simulink model "MCVT_Integration"  (R2020a)
settings: Solver=ode8  StartTime=0.0  StopTime=T_end  FixedStep=dt  RelTol=1e-3  EnableMultiTasking=on
values from neighbouring files (symbols this model uses):
  assumed_power_voltage = 1.25e2    (= 125)    [MCVT_Input_File.m]    (also in MCVT_Run_QuickCheck.m)
  battery_power_toggle = 1    [MCVT_Input_File.m]    (also in MCVT_Run_QuickCheck.m)
  battery_trickle_charge_param = 0.1    [MCVT_Input_File.m]    (also in MCVT_Run_QuickCheck.m)
  battery_trickle_leakage_param = 0.01    [MCVT_Input_File.m]    (also in MCVT_Run_QuickCheck.m)
  damage_case_id = 3    [MCVT_Input_File.m]    (also in MCVT_Run_QuickCheck.m = 1)
  dt = 1/fs    (= 0.001)    [MCVT_Run_QuickCheck.m]    (also in MCVT_Sim_Set.m)
  dt_2 = 1/fs_2    (= 0.001)    [MCVT_Run_QuickCheck.m]    (also in MCVT_Sim_Set.m)
  dt_3 = 1/fs_3    (= 0.001)    [MCVT_Run_QuickCheck.m]    (also in MCVT_Sim_Set.m)
  dt_5 = 1/fs_5    (= 0.005)    [MCVT_Run_QuickCheck.m]    (also in MCVT_Sim_Set.m)
  dt_8 = 1/fs_8    (= 0.05)    [MCVT_Run_QuickCheck.m]    (also in MCVT_Sim_Set.m)
  dt_9 = 1/fs_9    (= 0.001)    [MCVT_Run_QuickCheck.m]    (also in MCVT_Sim_Set.m)
  dust_met_duration = 3    [MCVT_Input_File.m]    (also in MCVT_Run_QuickCheck.m = 2)
  ECLSS_indoor_set_temperature = 298.15    [MCVT_Input_File.m]    (also in MCVT_Run_QuickCheck.m)
  energy_store_max_o = 150    [MCVT_Input_File.m]    (also in MCVT_Run_QuickCheck.m)
  energy_store_o = 120    [MCVT_Input_File.m]    (also in MCVT_Run_QuickCheck.m)
  fs = 1000    [MCVT_Run_QuickCheck.m]    (also in MCVT_Sim_Set.m)
  gain_charge_param = 1    [MCVT_Input_File.m]    (also in MCVT_Run_QuickCheck.m)
  ground_temperature = 373    [MCVT_Habitat_Design_Input_File.m]    (also in MCVT_Run_QuickCheck.m)
  I_max_charge = 800    [MCVT_Input_File.m]    (also in MCVT_Run_QuickCheck.m)
  I_max_discharge = 800    [MCVT_Input_File.m]    (also in MCVT_Run_QuickCheck.m)
  initial_pressure = 1e5    (= 100000)    [MCVT_Input_File.m]    (also in MCVT_Run_QuickCheck.m)
  initial_temperature = 295    [MCVT_Input_File.m]    (also in MCVT_Run_QuickCheck.m)
  interior_leak_rate = 0.00415    [MCVT_Input_File.m]    (also in MCVT_Run_QuickCheck.m)
  launch_dust_accum_start_time = 10    [MCVT_Input_File.m]    (also in MCVT_Run_QuickCheck.m = 2)
  launch_dust_accum_stop_time = 12    [MCVT_Input_File.m]    (also in MCVT_Run_QuickCheck.m = 3)
  meteorite_impact_time = 2    [MCVT_Input_File.m]    (also in MCVT_Run_QuickCheck.m = 1)
  nuclear_power_toggle = 1    [MCVT_Input_File.m]    (also in MCVT_Run_QuickCheck.m)
  print_interval = 30    [MCVT_Input_File.m]
  SOC_lower_thres = 0.5    [MCVT_Input_File.m]    (also in MCVT_Run_QuickCheck.m)
  SOC_upper_thres = 0.9    [MCVT_Input_File.m]    (also in MCVT_Run_QuickCheck.m)
  solar_power_toggle = 1    [MCVT_Input_File.m]    (also in MCVT_Run_QuickCheck.m)
  space_temperature = 4    [MCVT_Habitat_Design_Input_File.m]    (also in MCVT_Run_QuickCheck.m)
  Start_angle = 90    [MCVT_Input_File.m]    (also in MCVT_Run_QuickCheck.m)
undefined symbols (not defined in the file or in its folder): A_flow_restrictor, ACTIVITY_LOOKUP_TABLE, air, Aref, Bmap, Bmap2, Bmap3, comms, DiscIntNeverNeededParam, DmgSPL, dust_parameters, ECLSS_dust_damage_repair_time, ECLSS_heater_capacity, ECLSS_IPS_restrictor, ECLSS_IPS_setpointPressure, ECLSS_paint_damage_repair_time, ECLSS_radiatorLoop_discreteNumber, ECLSS_Vst, emptyActivity, expected_heat_add, FI, habitat, HIEM2SMM, HIEM2STM, HIEMDamageElements, impact_numbering, initial_SPLT_damage_index, input_air, input_Cond, input_CP, input_discretization, input_EV, input_Evap, input_radiatorLoop, K_ECLSS_dust_single, K_ECLSS_paint_single, K_Structure_damage_single, Kref, kW_to_W, meteoroidDustDep, mFRF, ndelays, nominalDustDep, noverlap, nuclear_toggle_gain, output_limits, panel_paint0, Ps0, reference_temperature, RepairTime_ECLSS_factor, Sensor_Location_SMM, SMM_T0, SMM_T1, SMM_T2, SMM_T3, SMM_T4, SMM_T4_0, SMM_T4_1, SMM_T4_2, solar_FDD_K ... (+73)
libraries: neural x350, simulink x39, xpcutilitieslib x7, xpcrtudplib x7, dspstat3 x1
(part 6 of 43)

== system MCVT_Integration/Sys2 - Structural System/Sys2_Structural_System/Structural Thermal Model 2.2/STM Temperature Sensors/ Resistance Temperature Detector2 ==
  Physical Interdependency - IN : Inport
  Switch Control : Inport  Port=2
  Power_Supply (kW) : Inport  Port=3
  Power Consumption : MATLAB Function  TreatAsAtomicUnit=on
  R = U/I : Gain  Gain=1/0.001
  R vs T : MATLAB Function  TreatAsAtomicUnit=on
  RTD with Self-heat Error : Subsystem
  RTD without Self-heat Error : Subsystem
  Self-heat Calculation : Subsystem
  Switch ON/OFF Control Block : Subsystem
  T vs R : MATLAB Function  TreatAsAtomicUnit=on
  Physical Interdependency - OUT : Outport  VectorParamsAs1DForOutWhenUnconnected=off
  Cyber Interdependency - OUT : Outport  Port=2  VectorParamsAs1DForOutWhenUnconnected=off
  Power_Usage (kW) : Outport  Port=3
  wires:
  T vs R/1(R) -> RTD without Self-heat Error/1(Resistance Change without Error), Self-heat Calculation/2(Resistance Change without Error)
  R vs T/1(T) -> Cyber Interdependency - OUT/1
  RTD with Self-heat Error/1(Voltage Output with Error) -> R = U/I/1
  Self-heat Calculation/1(Resistance Change with Error) -> RTD with Self-heat Error/1(Resistance Change with Error)
  R = U/I/1 -> R vs T/1(R)
  RTD without Self-heat Error/1(Current in Circuit) -> Self-heat Calculation/3(Current in Circuit)
  Switch Control/1 -> Switch ON/OFF Control Block/1(In1)
  Physical Interdependency - IN/1 -> Physical Interdependency - OUT/1, Switch ON/OFF Control Block/2(In2)
  Power Consumption/1(kWUsage3) -> Power_Usage (kW)/1
  Switch ON/OFF Control Block/1(Out1) -> Power Consumption/2(Switch), T vs R/1(T), Self-heat Calculation/1(Real Temperature)
  Power_Supply (kW)/1 -> Power Consumption/1(Supply)

== system MCVT_Integration/Sys3 - Power System/Power Generation Subsystem1/Nuclear Power Generation/SP-100 Power Generation/	Power Generation ==
  Disturbance : Inport
  Sub3_In_Dust_Accumulation (mg/cm^2) : Inport  Port=2
  Interpolation : Subsystem
  Nominal Power : Subsystem
  Power Generation : Subsystem
  Sensor Models : Subsystem
  Cyber Output SP100 : Outport
  Physical Output SP100 : Outport  Port=2
  Heat Rejection Fraction of Nominal : Outport  Port=3
  Measured Temp Values Nominal (K) : Outport  Port=4
  Power nominal (kW) : Outport  Port=5
  wires:
  [cyb_output1] -> Cyber Output SP100/1
  Disturbance/1 -> [disturbance]
  Power Generation/1(Physical Output) -> [phys_out]
  [phys_out1] -> Physical Output SP100/1
  Sub3_In_Dust_Accumulation (mg/cm^2)/1 -> [dust_accum4]
  [phys_input] -> Sensor Models/1(Physical Input)
  [tc1_interp] -> Sensor Models/3(tc1)
  [power_interp] -> Sensor Models/2(power (kW))
  [temp_flux] -> Interpolation/2(temp flux)
  [HRF_nom] -> Heat Rejection Fraction of Nominal/1
  [flow] -> Nominal Power/3(flow)
  [meas_nom] -> Measured Temp Values Nominal (K)/1
  [nom_vals] -> Nominal Power/1(nominal values)
  [power_nom1] -> Power nominal (kW)/1
  [disturbance] -> Power Generation/1(Disturbance Input)
  [dust_accum4] -> Power Generation/2(Sub3_In_Dust_Accumulation (mg/cm^2))
  [phys_out] -> Interpolation/1(Physical Output)
  Power Generation/2(temp flux) -> [temp_flux]
  [temp_flux] -> Nominal Power/2(temp flux)
  Power Generation/3(Heat Rejection Fraction of Nominal) -> [HRF_nom]
  Power Generation/4(flow) -> [flow]
  Power Generation/5(nominal values) -> [nom_vals]
  Nominal Power/1(Measured Temp Values Nominal (K)) -> [meas_nom]
  Nominal Power/2(power nominal (kW)) -> [power_nom1]
  Sensor Models/1(Cyber Output) -> [cyb_output1]
  Sensor Models/2(Physical Output SP100) -> [phys_out1]
  Interpolation/1(Physical Input) -> [phys_input]
  Interpolation/2(Tc1_interp (K)) -> [tc1_interp]
  Interpolation/3(Power_interp (kW)) -> [power_interp]

== system MCVT_Integration/Sys3 - Power System/Power Generation Subsystem1/Nuclear Power Generation/SP-100 Power Generation/FDD Iterative Online Residual Analysis1 ==
  Physical Output SP100 : Inport
  power nominal (kW) : Inport  Port=2
  Sub3_In_Intervention : Inport  Port=3
  " Digital Twin of SP100" : Subsystem
  Cyber Output : Outport
  wires:
  " Digital Twin of SP100"/1(Cyber Output FDD) -> Cyber Output/1
  power nominal (kW)/1 -> " Digital Twin of SP100"/2(power nominal (kW))
  Physical Output SP100/1 -> " Digital Twin of SP100"/1(Physical Output SP100)
  Sub3_In_Intervention/1 -> " Digital Twin of SP100"/3(Sub3_In_Intervention)

== system MCVT_Integration/Sys3 - Power System/Power Generation Subsystem1/Power Distribution and Storage System/Subsystem2/Dummy Electrical Power Consuming Equipment3 ==
  XX_In_Power_Available : Inport
  MATLAB Function : MATLAB Function  TreatAsAtomicUnit=on
  Power Draw Desired : Memory  InitialCondition=50
  p_3_desired : Outport
  wires:
  Power Draw Desired/1 -> p_3_desired/1
  MATLAB Function/1(p_desired) -> Power Draw Desired/1
  XX_In_Power_Available/1 -> MATLAB Function/1(p_avail)

== system MCVT_Integration/Sys8 - Interior Environment/Habitat Interior Environment Model/Temperature Sensors/ Resistance Temperature Detector/RTD with Self-heat Error ==
  Resistance Change with Error : Inport
  " Current in Circuit" : Constant  Value=0.001
  MATLAB Function : MATLAB Function  TreatAsAtomicUnit=on
  Voltage Output with Error : Outport  VectorParamsAs1DForOutWhenUnconnected=off
  wires:
  Resistance Change with Error/1 -> MATLAB Function/2(R)
  MATLAB Function/1(U) -> Voltage Output with Error/1
  " Current in Circuit"/1 -> MATLAB Function/1(I)

== system MCVT_Integration/Sys8 - Interior Environment/Habitat Interior Environment Model/Temperature Sensors/ Resistance Temperature Detector/RTD without Self-heat Error ==
  Resistance Change without Error : Inport
  " Current in Circuit" : Constant  Value=0.001
  MATLAB Function : MATLAB Function  TreatAsAtomicUnit=on
  Current in Circuit : Outport  VectorParamsAs1DForOutWhenUnconnected=off
  Voltage Output without Error : Outport  Port=2  VectorParamsAs1DForOutWhenUnconnected=off
  wires:
  Resistance Change without Error/1 -> MATLAB Function/2(R)
  MATLAB Function/2(U) -> Voltage Output without Error/1
  MATLAB Function/1(I) -> Current in Circuit/1
  " Current in Circuit"/1 -> MATLAB Function/1("I ")

== system MCVT_Integration/Sys8 - Interior Environment/Habitat Interior Environment Model/Temperature Sensors/ Resistance Temperature Detector/Self-heat Calculation ==
  note: Power = I 2 *R
  Real Temperature : Inport
  Resistance Change without Error : Inport  Port=2
  Current in Circuit : Inport  Port=3
  "  T vs R " : MATLAB Function  TreatAsAtomicUnit=on
  Add1 : Sum
  Divide1 : Product  Inputs=*/
  Gain : Gain  Gain=1000
  Product : Product
  Self Heating Constant (mW/℃) : Constant  Value=50
  Square : Math  Operator=square  SignedPower=on  SaturateOnIntegerOverflow=on
  Resistance Change with Error : Outport  VectorParamsAs1DForOutWhenUnconnected=off
  Self-heat Error : Outport  Port=2  VectorParamsAs1DForOutWhenUnconnected=off
  wires:
  Self Heating Constant (mW/℃)/1 -> Divide1/2
  Product/1 -> Divide1/1
  Add1/1 -> "  T vs R "/1(T)
  Divide1/1 -> Self-heat Error/1, Add1/2
  Resistance Change without Error/1 -> Product/1
  "  T vs R "/1(R) -> Resistance Change with Error/1
  Real Temperature/1 -> Add1/1
  Gain/1 -> Square/1
  Square/1 -> Product/2
  Current in Circuit/1 -> Gain/1

== system MCVT_Integration/Sys8 - Interior Environment/Habitat Interior Environment Model/Temperature Sensors/ Resistance Temperature Detector/Switch ON//OFF Control Block ==
  In1 : Inport
  In2 : Inport  Port=2
  Multiport Switch1 : MultiPortSwitch  Inputs=2  DiagnosticForDefault=None
  Switch OFF : Subsystem
  Switch ON : Subsystem
  Out1 : Outport  VectorParamsAs1DForOutWhenUnconnected=off
  wires:
  Multiport Switch1/1 -> Out1/1
  In2/1 -> Switch ON/1(In1), Switch OFF/1(In1)
  In1/1 -> Multiport Switch1/1
  Switch ON/1(ON) -> Multiport Switch1/2
  Switch OFF/1(OFF) -> Multiport Switch1/3

== system MCVT_Integration/Sys8 - Interior Environment/Habitat Interior Environment Model/Temperature Sensors/ Resistance Temperature Detector1/RTD with Self-heat Error ==
  Resistance Change with Error : Inport
  " Current in Circuit" : Constant  Value=0.001
  MATLAB Function : MATLAB Function  TreatAsAtomicUnit=on
  Voltage Output with Error : Outport  VectorParamsAs1DForOutWhenUnconnected=off
  wires:
  " Current in Circuit"/1 -> MATLAB Function/1(I)
  MATLAB Function/1(U) -> Voltage Output with Error/1
  Resistance Change with Error/1 -> MATLAB Function/2(R)

== system MCVT_Integration/Sys8 - Interior Environment/Habitat Interior Environment Model/Temperature Sensors/ Resistance Temperature Detector1/RTD without Self-heat Error ==
  Resistance Change without Error : Inport
  " Current in Circuit" : Constant  Value=0.001
  MATLAB Function : MATLAB Function  TreatAsAtomicUnit=on
  Current in Circuit : Outport  VectorParamsAs1DForOutWhenUnconnected=off
  Voltage Output without Error : Outport  Port=2  VectorParamsAs1DForOutWhenUnconnected=off
  wires:
  " Current in Circuit"/1 -> MATLAB Function/1("I ")
  MATLAB Function/1(I) -> Current in Circuit/1
  MATLAB Function/2(U) -> Voltage Output without Error/1
  Resistance Change without Error/1 -> MATLAB Function/2(R)

== system MCVT_Integration/Sys8 - Interior Environment/Habitat Interior Environment Model/Temperature Sensors/ Resistance Temperature Detector1/Self-heat Calculation ==
  note: Power = I 2 *R
  Real Temperature : Inport
  Resistance Change without Error : Inport  Port=2
  Current in Circuit : Inport  Port=3
  "  T vs R " : MATLAB Function  TreatAsAtomicUnit=on
  Add1 : Sum
  Divide1 : Product  Inputs=*/
  Gain : Gain  Gain=1000
  Product : Product
  Self Heating Constant (mW/℃) : Constant  Value=50
  Square : Math  Operator=square  SignedPower=on  SaturateOnIntegerOverflow=on
  Resistance Change with Error : Outport  VectorParamsAs1DForOutWhenUnconnected=off
  Self-heat Error : Outport  Port=2  VectorParamsAs1DForOutWhenUnconnected=off
  wires:
  Current in Circuit/1 -> Gain/1
  Square/1 -> Product/2
  Gain/1 -> Square/1
  Real Temperature/1 -> Add1/1
  "  T vs R "/1(R) -> Resistance Change with Error/1
  Resistance Change without Error/1 -> Product/1
  Divide1/1 -> Add1/2, Self-heat Error/1
  Add1/1 -> "  T vs R "/1(T)
  Product/1 -> Divide1/1
  Self Heating Constant (mW/℃)/1 -> Divide1/2

== system MCVT_Integration/Sys8 - Interior Environment/Habitat Interior Environment Model/Temperature Sensors/ Resistance Temperature Detector1/Switch ON//OFF Control Block ==
  In1 : Inport
  In2 : Inport  Port=2
  Multiport Switch1 : MultiPortSwitch  Inputs=2  DiagnosticForDefault=None
  Switch OFF : Subsystem
  Switch ON : Subsystem
  Out1 : Outport  VectorParamsAs1DForOutWhenUnconnected=off
  wires:
  Multiport Switch1/1 -> Out1/1
  In2/1 -> Switch ON/1(In1), Switch OFF/1(In1)
  In1/1 -> Multiport Switch1/1
  Switch ON/1(ON) -> Multiport Switch1/2
  Switch OFF/1(OFF) -> Multiport Switch1/3

== system MCVT_Integration/Sys8 - Interior Environment/Habitat Interior Environment Model/Temperature Sensors/ Resistance Temperature Detector2/RTD with Self-heat Error ==
  Resistance Change with Error : Inport
  " Current in Circuit" : Constant  Value=0.001
  MATLAB Function : MATLAB Function  TreatAsAtomicUnit=on
  Voltage Output with Error : Outport  VectorParamsAs1DForOutWhenUnconnected=off
  wires:
  Resistance Change with Error/1 -> MATLAB Function/2(R)
  MATLAB Function/1(U) -> Voltage Output with Error/1
  " Current in Circuit"/1 -> MATLAB Function/1(I)

== system MCVT_Integration/Sys8 - Interior Environment/Habitat Interior Environment Model/Temperature Sensors/ Resistance Temperature Detector2/RTD without Self-heat Error ==
  Resistance Change without Error : Inport
  " Current in Circuit" : Constant  Value=0.001
  MATLAB Function : MATLAB Function  TreatAsAtomicUnit=on
  Current in Circuit : Outport  VectorParamsAs1DForOutWhenUnconnected=off
  Voltage Output without Error : Outport  Port=2  VectorParamsAs1DForOutWhenUnconnected=off
  wires:
  Resistance Change without Error/1 -> MATLAB Function/2(R)
  MATLAB Function/2(U) -> Voltage Output without Error/1
  MATLAB Function/1(I) -> Current in Circuit/1
  " Current in Circuit"/1 -> MATLAB Function/1("I ")

== system MCVT_Integration/Sys8 - Interior Environment/Habitat Interior Environment Model/Temperature Sensors/ Resistance Temperature Detector2/Self-heat Calculation ==
  note: Power = I 2 *R
  Real Temperature : Inport
  Resistance Change without Error : Inport  Port=2
  Current in Circuit : Inport  Port=3
  "  T vs R " : MATLAB Function  TreatAsAtomicUnit=on
  Add1 : Sum
  Divide1 : Product  Inputs=*/
  Gain : Gain  Gain=1000
  Product : Product
  Self Heating Constant (mW/℃) : Constant  Value=50
  Square : Math  Operator=square  SignedPower=on  SaturateOnIntegerOverflow=on
  Resistance Change with Error : Outport  VectorParamsAs1DForOutWhenUnconnected=off
  Self-heat Error : Outport  Port=2  VectorParamsAs1DForOutWhenUnconnected=off
  wires:
  Self Heating Constant (mW/℃)/1 -> Divide1/2
  Product/1 -> Divide1/1
  Add1/1 -> "  T vs R "/1(T)
  Divide1/1 -> Self-heat Error/1, Add1/2
  Resistance Change without Error/1 -> Product/1
  "  T vs R "/1(R) -> Resistance Change with Error/1
  Real Temperature/1 -> Add1/1
  Gain/1 -> Square/1
  Square/1 -> Product/2
  Current in Circuit/1 -> Gain/1

== system MCVT_Integration/Sys8 - Interior Environment/Habitat Interior Environment Model/Temperature Sensors/ Resistance Temperature Detector2/Switch ON//OFF Control Block ==
  In1 : Inport
  In2 : Inport  Port=2
  Multiport Switch1 : MultiPortSwitch  Inputs=2  DiagnosticForDefault=None
  Switch OFF : Subsystem
  Switch ON : Subsystem
  Out1 : Outport  VectorParamsAs1DForOutWhenUnconnected=off
  wires:
  Multiport Switch1/1 -> Out1/1
  In2/1 -> Switch ON/1(In1), Switch OFF/1(In1)
  In1/1 -> Multiport Switch1/1
  Switch ON/1(ON) -> Multiport Switch1/2
  Switch OFF/1(OFF) -> Multiport Switch1/3

== system MCVT_Integration/Sys2 - Structural System/Sys2_Structural_System/Fault Detection and Diagnosis (FDD) 2.3/Accelerometer/Accelerometer/Accelerometer ==
  In1 : Inport
  Add : Sum
  Add1 : Sum
  Band-Limited White Noise : simulink/Sources/Band-Limited White Noise  Cov=Noise_power  Ts=Time_step  seed=10000
  Bias Voltage : Constant  Value=12
  Gain : Gain  Gain=1/1000
  Maximum/minimum Output Voltage : MATLAB Function  TreatAsAtomicUnit=on
  "Sensitivity " : Gain  Gain=1/1000
  Subtract : Sum  Inputs=+-
  Out : Outport  VectorParamsAs1DForOutWhenUnconnected=off
  wires:
  Gain/1 -> Add1/2
  In1/1 -> "Sensitivity "/1
  Maximum/minimum Output Voltage/1(y) -> Add1/1, Subtract/2
  "Sensitivity "/1 -> Add/1
  Bias Voltage/1 -> Add/2
  Add/1 -> Maximum/minimum Output Voltage/1(u)
  Band-Limited White Noise/1 -> Gain/1
  Add1/1 -> Subtract/1, Out/1

== system MCVT_Integration/Sys2 - Structural System/Sys2_Structural_System/Fault Detection and Diagnosis (FDD) 2.3/Accelerometer/Accelerometer/Switch ON//OFF Control Block ==
  In1 : Inport
  In2 : Inport  Port=2
  In3 : Inport  Port=3
  Multiport Switch : MultiPortSwitch  Inputs=2  DataPortIndices={1,2,3,4}
  Switch OFF : Subsystem
  Switch ON : Subsystem
  Out1 : Outport  VectorParamsAs1DForOutWhenUnconnected=off
  wires:
  In3/1 -> Switch ON/1(In1), Switch OFF/2(All Acc)
  In2/1 -> Switch OFF/1(Sensor)
  Switch OFF/1(Selected Acc) -> Multiport Switch/3
  In1/1 -> Multiport Switch/1
  Switch ON/1(ON) -> Multiport Switch/2
  Multiport Switch/1 -> Out1/1

== system MCVT_Integration/Sys2 - Structural System/Sys2_Structural_System/Fault Detection and Diagnosis (FDD) 2.3/Pattern Recognition Neural Network/Pattern Recognition Neural Network with 10% Noise/Layer 1 ==
  "p{1}" : Inport  PortDimensions=2304
      mask Display: e = 0.06;c1 = [120 230 180]/255;c2 = ([120 230 180]+0)/(256+128);c3 = ([120 230 180]+128)/(256+128);patch([0 1 1 0],[0 0 1 1],c1);patch([0 1 1 1-e 1-e 0+e],[0 0 1 1-e 0+e 0+e],c2);patch([0 0 1 1-e 0+e 0+e],[0 1 1 1-e 1-e 0+e],c3);plot([0 1 1 0 0],[0 0 1 1 0]);
  Delays 1 : Subsystem
      mask Display: e = 0.06;c1 = [120 150 230]/255;c2 = ([120 150 230]+0)/383;c3 = ([120 150 230]+128)/383;patch([0 1 1 0],[0 0 1 1],c1);patch([0 1 1 1-e 1-e 0+e],[0 0 1 1-e 0+e 0+e],c2);patch([0 0 1 1-e 0+e 0+e],[0 1 1 1-e 1-e 0+e],c3);plot([0 1 1 0 0],[0 0 1 1 0]);disp('0');
  "IW{1,1}" : Subsystem
      mask Display: e = 0.06;c1 = [120 150 230]/255;c2 = ([120 150 230]+0)/383;c3 = ([120 150 230]+128)/383;patch([0 1 1 0],[0 0 1 1],c1);patch([0 1 1 1-e 1-e 0+e],[0 0 1 1-e 0+e 0+e],c2);patch([0 0 1 1-e 0+e 0+e],[0 1 1 1-e 1-e 0+e],c3);plot([0 1 1 0 0],[0 0 1 1 0]);disp('W');
  "b{1}" : Constant  Value=[-1.448111920363924554067125427536666393280029296875;1.2219145199634631193674749738420359790325164794921875;1.06138547882932687826951223541982471942901611328125;-1.0100920024028692267847873154096305370330810546875;0.79025279652121527274033496723859570920467376708984375;0.74966144854164895594550444002379663288593292236328125;0.51235342775193382092169258612557314336299896240234375;-0.300296253789623712737721916710142977535724639892578125;-0.150518681120877240164901422758703120052814483642578125;-0.04908487537234955400133884495517122559249401092529296875;-0.12749577645076903831267145505989901721477508544921875;0.237681886259743457845416969576035626232624053955078125;0.410109645742695538839228674987680278718471527099609375;0.55443039205315713946475852935691364109516143798828125;-0.624811371810401805504397998447529971599578857421875;-0.83385508908698613428356338772573508322238922119140625;0.98231480364563161788993284062598831951618194580078125;-1.1488024222274881847027927506132982671260833740234375;-1.0592228830060081978814423564472235739231109619140625;-1.4291108592006167565813257169793359935283660888671875]
      mask Display: e = 0.06;c1 = [120 150 230]/255;c2 = ([120 150 230]+0)/383;c3 = ([120 150 230]+128)/383;patch([0 1 1 0],[0 0 1 1],c1);patch([0 1 1 1-e 1-e 0+e],[0 0 1 1-e 0+e 0+e],c2);patch([0 0 1 1-e 0+e 0+e],[0 1 1 1-e 1-e 0+e],c3);plot([0 1 1 0 0],[0 0 1 1 0]);disp('b');
  netsum : Sum  Inputs=2  InputSameDT=on  SaturateOnIntegerOverflow=on  OutDataTypeStr=Inherit: Same as first input
      mask Display: e = 0.06;c1 = [120 150 230]/255;c2 = ([120 150 230]+0)/383;c3 = ([120 150 230]+128)/383;patch([0 1 1 0],[0 0 1 1],c1);patch([0 1 1 1-e 1-e 0+e],[0 0 1 1-e 0+e 0+e],c2);patch([0 0 1 1-e 0+e 0+e],[0 1 1 1-e 1-e 0+e],c3);plot([0 1 1 0 0],[0 0 1 1 0]);disp('+');
  tansig : neural/Transfer Functions/tansig
  "a{1}" : Outport  InitialOutput=[0;0;0;0;0;0;0;0;0;0;0;0;0;0;0;0;0;0;0;0]
      mask Display: e = 0.06;c1 = [120 230 180]/255;c2 = ([120 230 180]+0)/(256+128);c3 = ([120 230 180]+128)/(256+128);patch([0 1 1 0],[0 0 1 1],c1);patch([0 1 1 1-e 1-e 0+e],[0 0 1 1-e 0+e 0+e],c2);patch([0 0 1 1-e 0+e 0+e],[0 1 1 1-e 1-e 0+e],c3);plot([0 1 1 0 0],[0 0 1 1 0]);
  wires:
  "p{1}"/1 -> Delays 1/1("p{1}")
  Delays 1/1("pd{1,1}") -> "IW{1,1}"/1("pd{1,1}")
  "IW{1,1}"/1("iz{1,1}") -> netsum/1
  "b{1}"/1 -> netsum/2
  netsum/1 -> tansig/1
  tansig/1 -> "a{1}"/1

== system MCVT_Integration/Sys2 - Structural System/Sys2_Structural_System/Fault Detection and Diagnosis (FDD) 2.3/Pattern Recognition Neural Network/Pattern Recognition Neural Network with 10% Noise/Layer 2 ==
  "a{1} " : Inport  PortDimensions=20
      mask Display: e = 0.06;c1 = [120 230 180]/255;c2 = ([120 230 180]+0)/(256+128);c3 = ([120 230 180]+128)/(256+128);patch([0 1 1 0],[0 0 1 1],c1);patch([0 1 1 1-e 1-e 0+e],[0 0 1 1-e 0+e 0+e],c2);patch([0 0 1 1-e 0+e 0+e],[0 1 1 1-e 1-e 0+e],c3);plot([0 1 1 0 0],[0 0 1 1 0]);
  Delays 1 : Subsystem
      mask Display: e = 0.06;c1 = [120 150 230]/255;c2 = ([120 150 230]+0)/383;c3 = ([120 150 230]+128)/383;patch([0 1 1 0],[0 0 1 1],c1);patch([0 1 1 1-e 1-e 0+e],[0 0 1 1-e 0+e 0+e],c2);patch([0 0 1 1-e 0+e 0+e],[0 1 1 1-e 1-e 0+e],c3);plot([0 1 1 0 0],[0 0 1 1 0]);disp('0');
  "LW{2,1}" : Subsystem
      mask Display: e = 0.06;c1 = [120 150 230]/255;c2 = ([120 150 230]+0)/383;c3 = ([120 150 230]+128)/383;patch([0 1 1 0],[0 0 1 1],c1);patch([0 1 1 1-e 1-e 0+e],[0 0 1 1-e 0+e 0+e],c2);patch([0 0 1 1-e 0+e 0+e],[0 1 1 1-e 1-e 0+e],c3);plot([0 1 1 0 0],[0 0 1 1 0]);disp('W');
  "b{2}" : Constant  Value=[1.5522179970064200471568938155542127788066864013671875;-1.530189960539861449007048577186651527881622314453125;1.17737219934708914337306850939057767391204833984375;0.911249658526244399325833001057617366313934326171875;-0.79481990535408986175980317057110369205474853515625;0.82857262300878764538225595970288850367069244384765625;-0.63402312802043792050454840136808343231678009033203125;0.41511669727381772521113134644110687077045440673828125;0.331096381229687930503047255115234293043613433837890625;-0.1862115961904717564845412880458752624690532684326171875;-0.07959776477177264719653493330042692832648754119873046875;0.1625073919351583684456130640683113597333431243896484375;-0.4231075237093404606270041767857037484645843505859375;0.602313144747966600078825649688951671123504638671875;0.8769423608435726880117044856888242065906524658203125;0.93415228872039424157236453538644127547740936279296875;-1.1697472432610001025210522129782475531101226806640625;-1.3099537990230967210436574532650411128997802734375;-1.401738096984951109646999611868523061275482177734375;-1.4895027559078852785745539222261868417263031005859375]
      mask Display: e = 0.06;c1 = [120 150 230]/255;c2 = ([120 150 230]+0)/383;c3 = ([120 150 230]+128)/383;patch([0 1 1 0],[0 0 1 1],c1);patch([0 1 1 1-e 1-e 0+e],[0 0 1 1-e 0+e 0+e],c2);patch([0 0 1 1-e 0+e 0+e],[0 1 1 1-e 1-e 0+e],c3);plot([0 1 1 0 0],[0 0 1 1 0]);disp('b');
  netsum : Sum  Inputs=2  InputSameDT=on  SaturateOnIntegerOverflow=on  OutDataTypeStr=Inherit: Same as first input
      mask Display: e = 0.06;c1 = [120 150 230]/255;c2 = ([120 150 230]+0)/383;c3 = ([120 150 230]+128)/383;patch([0 1 1 0],[0 0 1 1],c1);patch([0 1 1 1-e 1-e 0+e],[0 0 1 1-e 0+e 0+e],c2);patch([0 0 1 1-e 0+e 0+e],[0 1 1 1-e 1-e 0+e],c3);plot([0 1 1 0 0],[0 0 1 1 0]);disp('+');
  tansig : neural/Transfer Functions/tansig
  "a{2}" : Outport  InitialOutput=[0;0;0;0;0;0;0;0;0;0;0;0;0;0;0;0;0;0;0;0]
      mask Display: e = 0.06;c1 = [120 230 180]/255;c2 = ([120 230 180]+0)/(256+128);c3 = ([120 230 180]+128)/(256+128);patch([0 1 1 0],[0 0 1 1],c1);patch([0 1 1 1-e 1-e 0+e],[0 0 1 1-e 0+e 0+e],c2);patch([0 0 1 1-e 0+e 0+e],[0 1 1 1-e 1-e 0+e],c3);plot([0 1 1 0 0],[0 0 1 1 0]);
  wires:
  "a{1} "/1 -> Delays 1/1("p{2}")
  Delays 1/1("pd{2,1}") -> "LW{2,1}"/1("ad{2,1}")
  "LW{2,1}"/1("lz{2,1}") -> netsum/1
  "b{2}"/1 -> netsum/2
  netsum/1 -> tansig/1
  tansig/1 -> "a{2}"/1

== system MCVT_Integration/Sys2 - Structural System/Sys2_Structural_System/Fault Detection and Diagnosis (FDD) 2.3/Pattern Recognition Neural Network/Pattern Recognition Neural Network with 10% Noise/Layer 3 ==
  "a{2} " : Inport  PortDimensions=20
      mask Display: e = 0.06;c1 = [120 230 180]/255;c2 = ([120 230 180]+0)/(256+128);c3 = ([120 230 180]+128)/(256+128);patch([0 1 1 0],[0 0 1 1],c1);patch([0 1 1 1-e 1-e 0+e],[0 0 1 1-e 0+e 0+e],c2);patch([0 0 1 1-e 0+e 0+e],[0 1 1 1-e 1-e 0+e],c3);plot([0 1 1 0 0],[0 0 1 1 0]);
  Delays 1 : Subsystem
      mask Display: e = 0.06;c1 = [120 150 230]/255;c2 = ([120 150 230]+0)/383;c3 = ([120 150 230]+128)/383;patch([0 1 1 0],[0 0 1 1],c1);patch([0 1 1 1-e 1-e 0+e],[0 0 1 1-e 0+e 0+e],c2);patch([0 0 1 1-e 0+e 0+e],[0 1 1 1-e 1-e 0+e],c3);plot([0 1 1 0 0],[0 0 1 1 0]);disp('0');
  "LW{3,2}" : Subsystem
      mask Display: e = 0.06;c1 = [120 150 230]/255;c2 = ([120 150 230]+0)/383;c3 = ([120 150 230]+128)/383;patch([0 1 1 0],[0 0 1 1],c1);patch([0 1 1 1-e 1-e 0+e],[0 0 1 1-e 0+e 0+e],c2);patch([0 0 1 1-e 0+e 0+e],[0 1 1 1-e 1-e 0+e],c3);plot([0 1 1 0 0],[0 0 1 1 0]);disp('W');
  "b{3}" : Constant  Value=[0.3788768286576897903472627149312756955623626708984375;-0.77561117274222157735863447669544257223606109619140625;-1.015921648978773017546473056427203118801116943359375;-0.81541846439209575425621778776985593140125274658203125;0.0715714946480312530496803447022102773189544677734375;0.8991230815736466208676347378059290349483489990234375]
      mask Display: e = 0.06;c1 = [120 150 230]/255;c2 = ([120 150 230]+0)/383;c3 = ([120 150 230]+128)/383;patch([0 1 1 0],[0 0 1 1],c1);patch([0 1 1 1-e 1-e 0+e],[0 0 1 1-e 0+e 0+e],c2);patch([0 0 1 1-e 0+e 0+e],[0 1 1 1-e 1-e 0+e],c3);plot([0 1 1 0 0],[0 0 1 1 0]);disp('b');
  netsum : Sum  Inputs=2  InputSameDT=on  SaturateOnIntegerOverflow=on  OutDataTypeStr=Inherit: Same as first input
      mask Display: e = 0.06;c1 = [120 150 230]/255;c2 = ([120 150 230]+0)/383;c3 = ([120 150 230]+128)/383;patch([0 1 1 0],[0 0 1 1],c1);patch([0 1 1 1-e 1-e 0+e],[0 0 1 1-e 0+e 0+e],c2);patch([0 0 1 1-e 0+e 0+e],[0 1 1 1-e 1-e 0+e],c3);plot([0 1 1 0 0],[0 0 1 1 0]);disp('+');
  softmax : neural/Transfer Functions/softmax
      settings: SourceProductBaseCode=NN
  "a{3}" : Outport  InitialOutput=[0;0;0;0;0;0]
      mask Display: e = 0.06;c1 = [120 230 180]/255;c2 = ([120 230 180]+0)/(256+128);c3 = ([120 230 180]+128)/(256+128);patch([0 1 1 0],[0 0 1 1],c1);patch([0 1 1 1-e 1-e 0+e],[0 0 1 1-e 0+e 0+e],c2);patch([0 0 1 1-e 0+e 0+e],[0 1 1 1-e 1-e 0+e],c3);plot([0 1 1 0 0],[0 0 1 1 0]);
  wires:
  "a{2} "/1 -> Delays 1/1("p{3}")
  Delays 1/1("pd{3,2}") -> "LW{3,2}"/1("ad{3,2}")
  "LW{3,2}"/1("lz{3,2}") -> netsum/1
  "b{3}"/1 -> netsum/2
  netsum/1 -> softmax/1
  softmax/1 -> "a{3}"/1
